simulink model "MX_Gateway"  (Simulink 9.0)
settings: Solver=FixedStepDiscrete  StartTime=0.0  StopTime=100  FixedStep=0.01  RelTol=1e-3
values from neighbouring files (symbols this model uses):
  ACTIVE = Bobcat.Parameter; CreateDefine( ACTIVE                         ,1                          ,'Define' ,'defines.h' )    [defines.m]
  ACTIVE_MODE_ONE = Bobcat.Parameter; CreateDefine( ACTIVE_MODE_ONE                ,19                         ,'Define' ,'defines.h' )    [defines.m]
  AUTHENTICATED = Bobcat.Parameter; CreateDefine( AUTHENTICATED                  ,1                          ,'Define' ,'defines.h' )    [defines.m]
  AUX_EMEA_CONTROLS = Bobcat.Parameter; CreateDefine( AUX_EMEA_CONTROLS  ,1   ,'Define' ,'defines.h' )    [enums.m]
  AUX_FLOW_HIGH = Bobcat.Parameter; CreateDefine( AUX_FLOW_HIGH           ,4  ,'Define' ,'defines.h' )    [enums.m]
  AUX_FLOW_INACTIVE = Bobcat.Parameter; CreateDefine( AUX_FLOW_INACTIVE       ,0  ,'Define' ,'defines.h' )    [enums.m]
  AUX_FLOW_LOW = Bobcat.Parameter; CreateDefine( AUX_FLOW_LOW            ,1  ,'Define' ,'defines.h' )    [enums.m]
  AUX_FLOW_MEDIUM = Bobcat.Parameter; CreateDefine( AUX_FLOW_MEDIUM         ,2  ,'Define' ,'defines.h' )    [enums.m]
  AUX_FLOW_STANDARD = Bobcat.Parameter; CreateDefine( AUX_FLOW_STANDARD       ,3  ,'Define' ,'defines.h' )    [enums.m]
  AUX_NON_FUNCTIONAL = Bobcat.Parameter; CreateDefine( AUX_NON_FUNCTIONAL ,255 ,'Define' ,'defines.h' )    [enums.m]
  BASE_ACTIVE = Bobcat.Parameter; CreateDefine( BASE_ACTIVE                    ,253                        ,'Define' ,'defines.h' )    [defines.m]
  BASE_DETENT = Bobcat.Parameter; CreateDefine( BASE_DETENT                    ,1                          ,'Define' ,'defines.h' )    [defines.m]
  BEEP_NONE = Bobcat.Parameter; CreateDefine( BEEP_NONE                      ,255                        ,'Define' ,'defines.h' )    [defines.m]
  CD_AIR_FILTER_DEBOUNCE_NORMAL = Bobcat.Parameter; CreateAliasCD( CD_AIR_FILTER_DEBOUNCE_NORMAL                        ,uint16(500)                ,'ConstantDataPointer' ,'CONSTANT_DATA' ,'AIR_FILTER_DEBOUNCE_NORMAL')    [cdata.m]    (also in hc12_cdata.m; m4_cdata.m)
  CD_AIR_FILTER_DEBOUNCE_NOT_CONNECTED = Bobcat.Parameter; CreateAliasCD( CD_AIR_FILTER_DEBOUNCE_NOT_CONNECTED                 ,uint16(3000)               ,'ConstantDataPointer' ,'CONSTANT_DATA' ,'AIR_FILTER_DEBOUNCE_NOT_CONNECTED')    [cdata.m]    (also in hc12_cdata.m; m4_cdata.m)
  CD_AIR_FILTER_DEBOUNCE_PLUGGED = Bobcat.Parameter; CreateAliasCD( CD_AIR_FILTER_DEBOUNCE_PLUGGED                       ,uint16(3000)               ,'ConstantDataPointer' ,'CONSTANT_DATA' ,'AIR_FILTER_DEBOUNCE_PLUGGED')    [cdata.m]    (also in hc12_cdata.m; m4_cdata.m)
  CD_AIR_FILTER_FUNCTION = Bobcat.Parameter; CreateAliasCD( CD_AIR_FILTER_FUNCTION                               ,uint8(255)                 ,'ConstantDataPointer' ,'CONSTANT_DATA' ,'AIR_FILTER_FUNCTION')    [cdata.m]    (also in hc12_cdata.m; m4_cdata.m)
  CD_AIR_FILTER_PLUGGED_WEIGHT = Bobcat.Parameter; CreateAliasCD( CD_AIR_FILTER_PLUGGED_WEIGHT                         ,uint8(2)                   ,'ConstantDataPointer' ,'CONSTANT_DATA' ,'AIR_FILTER_PLUGGED_WEIGHT')    [cdata.m]    (also in hc12_cdata.m; m4_cdata.m)
  CD_AIR_FILTER_UNPLUGGED_WEIGHT = Bobcat.Parameter; CreateAliasCD( CD_AIR_FILTER_UNPLUGGED_WEIGHT                       ,uint8(1)                   ,'ConstantDataPointer' ,'CONSTANT_DATA' ,'AIR_FILTER_UNPLUGGED_WEIGHT')    [cdata.m]    (also in hc12_cdata.m; m4_cdata.m)
  CD_AUTOIDLE_ARMBAR_DELAY = Bobcat.Parameter; CreateAliasCD( CD_AUTOIDLE_ARMBAR_DELAY                             ,uint8(2)                   ,'ConstantDataPointer' ,'CONSTANT_DATA' ,'AUTOIDLE_ARMBAR_DELAY')    [cdata.m]    (also in hc12_cdata.m; m4_cdata.m)
  CD_AUTOIDLE_FUNCTION = Bobcat.Parameter; CreateAliasCD( CD_AUTOIDLE_FUNCTION                                 ,uint8(255)                 ,'ConstantDataPointer' ,'CONSTANT_DATA' ,'AUTOIDLE_FUNCTION')    [cdata.m]    (also in hc12_cdata.m; m4_cdata.m)
  CD_AUTOIDLE_IDLE_DELAY = Bobcat.Parameter; CreateAliasCD( CD_AUTOIDLE_IDLE_DELAY                               ,uint8(40)                  ,'ConstantDataPointer' ,'CONSTANT_DATA' ,'AUTOIDLE_IDLE_DELAY')    [cdata.m]    (also in hc12_cdata.m; m4_cdata.m)
  CD_AUTOIDLE_LOW_IDLE_START = Bobcat.Parameter; CreateAliasCD( CD_AUTOIDLE_LOW_IDLE_START                           ,uint8(255)                 ,'ConstantDataPointer' ,'CONSTANT_DATA' ,'AUTOIDLE_LOW_IDLE_START')    [cdata.m]    (also in hc12_cdata.m; m4_cdata.m)
  CD_AUTOIDLE_PRESSURE_OFF = Bobcat.Parameter; CreateAliasCD( CD_AUTOIDLE_PRESSURE_OFF                             ,uint16(300)                ,'ConstantDataPointer' ,'CONSTANT_DATA' ,'AUTOIDLE_PRESSURE_OFF')    [cdata.m]    (also in hc12_cdata.m; m4_cdata.m)
  CD_AUTOIDLE_PRESSURE_ON = Bobcat.Parameter; CreateAliasCD( CD_AUTOIDLE_PRESSURE_ON                              ,uint16(270)                ,'ConstantDataPointer' ,'CONSTANT_DATA' ,'AUTOIDLE_PRESSURE_ON')    [cdata.m]    (also in hc12_cdata.m; m4_cdata.m)
  CD_AUTOSHIFT_FUNCTION = Bobcat.Parameter; CreateAliasCD( CD_AUTOSHIFT_FUNCTION                                ,uint8(255)                 ,'ConstantDataPointer' ,'CONSTANT_DATA' ,'AUTOSHIFT_FUNCTION')    [cdata.m]    (also in hc12_cdata.m; m4_cdata.m)
  CD_AUTOSHIFT_PHIGH1 = Bobcat.Parameter; CreateAliasCD( CD_AUTOSHIFT_PHIGH1                                  ,uint16(205)                ,'ConstantDataPointer' ,'CONSTANT_DATA' ,'AUTOSHIFT_PHIGH1')    [cdata.m]    (also in hc12_cdata.m; m4_cdata.m)
  CD_AUTOSHIFT_PHIGH2 = Bobcat.Parameter; CreateAliasCD( CD_AUTOSHIFT_PHIGH2                                  ,uint16(193)                ,'ConstantDataPointer' ,'CONSTANT_DATA' ,'AUTOSHIFT_PHIGH2')    [cdata.m]    (also in hc12_cdata.m; m4_cdata.m)
  CD_AUTOSHIFT_PHIGH_TEMP1 = Bobcat.Parameter; CreateAliasCD( CD_AUTOSHIFT_PHIGH_TEMP1                             ,int16(70)                  ,'ConstantDataPointer' ,'CONSTANT_DATA' ,'AUTOSHIFT_PHIGH_TEMP1')    [cdata.m]    (also in hc12_cdata.m; m4_cdata.m)
  CD_AUTOSHIFT_PHIGH_TEMP2 = Bobcat.Parameter; CreateAliasCD( CD_AUTOSHIFT_PHIGH_TEMP2                             ,int16(160)                 ,'ConstantDataPointer' ,'CONSTANT_DATA' ,'AUTOSHIFT_PHIGH_TEMP2')    [cdata.m]    (also in hc12_cdata.m; m4_cdata.m)
  CD_AUTOSHIFT_PLOW1 = Bobcat.Parameter; CreateAliasCD( CD_AUTOSHIFT_PLOW1                                   ,uint16(130)                ,'ConstantDataPointer' ,'CONSTANT_DATA' ,'AUTOSHIFT_PLOW1')    [cdata.m]    (also in hc12_cdata.m; m4_cdata.m)
  CD_AUTOSHIFT_PLOW2 = Bobcat.Parameter; CreateAliasCD( CD_AUTOSHIFT_PLOW2                                   ,uint16(100)                ,'ConstantDataPointer' ,'CONSTANT_DATA' ,'AUTOSHIFT_PLOW2')    [cdata.m]    (also in hc12_cdata.m; m4_cdata.m)
  CD_AUTOSHIFT_PLOW_TEMP1 = Bobcat.Parameter; CreateAliasCD( CD_AUTOSHIFT_PLOW_TEMP1                              ,int16(20)                  ,'ConstantDataPointer' ,'CONSTANT_DATA' ,'AUTOSHIFT_PLOW_TEMP1')    [cdata.m]    (also in hc12_cdata.m; m4_cdata.m)
  CD_AUTOSHIFT_PLOW_TEMP2 = Bobcat.Parameter; CreateAliasCD( CD_AUTOSHIFT_PLOW_TEMP2                              ,int16(80)                  ,'ConstantDataPointer' ,'CONSTANT_DATA' ,'AUTOSHIFT_PLOW_TEMP2')    [cdata.m]    (also in hc12_cdata.m; m4_cdata.m)
  CD_AUTOSHIFT_TRAVEL_DELAY = Bobcat.Parameter; CreateAliasCD( CD_AUTOSHIFT_TRAVEL_DELAY                            ,uint8(32)                  ,'ConstantDataPointer' ,'CONSTANT_DATA' ,'AUTOSHIFT_TRAVEL_DELAY')    [cdata.m]    (also in hc12_cdata.m; m4_cdata.m)
  CD_AUX2_CRACKPOINT_CURRENT = Bobcat.Parameter; CreateAliasCD( CD_AUX2_CRACKPOINT_CURRENT                           ,fi(0,0,16,12,'hex','0c28') ,'ConstantDataPointer' ,'CONSTANT_DATA' ,'AUX2_CRACKPOINT_CURRENT')    [cdata.m]    (also in hc12_cdata.m; m4_cdata.m)
  CD_AUX2_FLOW_LOW_FUNCTION = Bobcat.Parameter; CreateAliasCD( CD_AUX2_FLOW_LOW_FUNCTION                            ,uint8(255)                 ,'ConstantDataPointer' ,'CONSTANT_DATA' ,'AUX2_FLOW_LOW_FUNCTION')    [cdata.m]    (also in hc12_cdata.m; m4_cdata.m)
  CD_AUX2_FLOW_MED_FUNCTION = Bobcat.Parameter; CreateAliasCD( CD_AUX2_FLOW_MED_FUNCTION                            ,uint8(255)                 ,'ConstantDataPointer' ,'CONSTANT_DATA' ,'AUX2_FLOW_MED_FUNCTION')    [cdata.m]    (also in hc12_cdata.m; m4_cdata.m)
  CD_AUX2_HI_FLOW_PERCENT = Bobcat.Parameter; CreateAliasCD( CD_AUX2_HI_FLOW_PERCENT                              ,fi(0,0,16,16,'hex','ffff') ,'ConstantDataPointer' ,'CONSTANT_DATA' ,'AUX2_HI_FLOW_PERCENT')    [cdata.m]    (also in hc12_cdata.m; m4_cdata.m)
  CD_AUX2_LOW_FLOW_PERCENT = Bobcat.Parameter; CreateAliasCD( CD_AUX2_LOW_FLOW_PERCENT                             ,fi(0,0,16,16,'hex','7333') ,'ConstantDataPointer' ,'CONSTANT_DATA' ,'AUX2_LOW_FLOW_PERCENT')    [cdata.m]    (also in hc12_cdata.m; m4_cdata.m)
  CD_AUX2_MAX_FLOW_CURRENT = Bobcat.Parameter; CreateAliasCD( CD_AUX2_MAX_FLOW_CURRENT                             ,fi(0,0,16,12,'hex','14cc') ,'ConstantDataPointer' ,'CONSTANT_DATA' ,'AUX2_MAX_FLOW_CURRENT')    [cdata.m]    (also in hc12_cdata.m; m4_cdata.m)
  CD_AUX2_MED_FLOW_PERCENT = Bobcat.Parameter; CreateAliasCD( CD_AUX2_MED_FLOW_PERCENT                             ,fi(0,0,16,16,'hex','9999') ,'ConstantDataPointer' ,'CONSTANT_DATA' ,'AUX2_MED_FLOW_PERCENT')    [cdata.m]    (also in hc12_cdata.m; m4_cdata.m)
  CD_AUX_CRACKPOINT_CURRENT = Bobcat.Parameter; CreateAliasCD( CD_AUX_CRACKPOINT_CURRENT                            ,fi(0,0,16,12,'hex','0000') ,'ConstantDataPointer' ,'CONSTANT_DATA' ,'AUX_CRACKPOINT_CURRENT')    [cdata.m]    (also in hc12_cdata.m; m4_cdata.m)
  CD_AUX_DETENT_FLASH_TIME = Bobcat.Parameter; CreateAliasCD( CD_AUX_DETENT_FLASH_TIME                             ,uint16(72)                 ,'ConstantDataPointer' ,'CONSTANT_DATA' ,'AUX_DETENT_FLASH_TIME')    [cdata.m]    (also in hc12_cdata.m; m4_cdata.m)
  CD_AUX_DETENT_HOLD_TIME = Bobcat.Parameter; CreateAliasCD( CD_AUX_DETENT_HOLD_TIME                              ,uint16(100)                ,'ConstantDataPointer' ,'CONSTANT_DATA' ,'AUX_DETENT_HOLD_TIME')    [cdata.m]    (also in hc12_cdata.m; m4_cdata.m)
  CD_AUX_FULL_ON_AUX1 = Bobcat.Parameter; CreateAliasCD( CD_AUX_FULL_ON_AUX1                                  ,fi(0,0,16,12,'hex','10cd') ,'ConstantDataPointer' ,'CONSTANT_DATA' ,'AUX_FULL_ON_AUX1')    [cdata.m]    (also in hc12_cdata.m; m4_cdata.m)
  CD_AUX_FULL_ON_AUX2 = Bobcat.Parameter; CreateAliasCD( CD_AUX_FULL_ON_AUX2                                  ,fi(0,0,16,12,'hex','139d') ,'ConstantDataPointer' ,'CONSTANT_DATA' ,'AUX_FULL_ON_AUX2')    [cdata.m]    (also in hc12_cdata.m; m4_cdata.m)
  CD_AUX_FULL_ON_AUX3 = Bobcat.Parameter; CreateAliasCD( CD_AUX_FULL_ON_AUX3                                  ,fi(0,0,16,12,'hex','17d7') ,'ConstantDataPointer' ,'CONSTANT_DATA' ,'AUX_FULL_ON_AUX3')    [cdata.m]    (also in hc12_cdata.m; m4_cdata.m)
  CD_AUX_FUNCTION = Bobcat.Parameter; CreateAliasCD( CD_AUX_FUNCTION                                      ,uint8(0)                   ,'ConstantDataPointer' ,'CONSTANT_DATA' ,'AUX_FUNCTION')    [cdata.m]    (also in hc12_cdata.m; m4_cdata.m)
  CD_AUX_RATE_LIMIT_CRACKPOINT = Bobcat.Parameter; CreateAliasCD( CD_AUX_RATE_LIMIT_CRACKPOINT                         ,fi(0,0,16,12,'hex','0800') ,'ConstantDataPointer' ,'CONSTANT_DATA' ,'AUX_RATE_LIMIT_CRACKPOINT')    [cdata.m]    (also in hc12_cdata.m; m4_cdata.m)
  CD_AUX_RATE_LIMIT_CRACKPOINT_RATE = Bobcat.Parameter; CreateAliasCD( CD_AUX_RATE_LIMIT_CRACKPOINT_RATE                    ,fi(0,1,32,12,'int',102400) ,'ConstantDataPointer' ,'CONSTANT_DATA' ,'AUX_RATE_LIMIT_CRACKPOINT_RATE')    [cdata.m]    (also in hc12_cdata.m; m4_cdata.m)
  CD_AUX_RATE_LIMIT_FUNCTION = Bobcat.Parameter; CreateAliasCD( CD_AUX_RATE_LIMIT_FUNCTION                           ,uint8(255)                 ,'ConstantDataPointer' ,'CONSTANT_DATA' ,'AUX_RATE_LIMIT_FUNCTION')    [cdata.m]    (also in hc12_cdata.m; m4_cdata.m)
  CD_AUX_RATE_LIMIT_MAX_ENG_SPD = Bobcat.Parameter; CreateAliasCD( CD_AUX_RATE_LIMIT_MAX_ENG_SPD                        ,uint16(1600)               ,'ConstantDataPointer' ,'CONSTANT_DATA' ,'AUX_RATE_LIMIT_MAX_ENG_SPD')    [cdata.m]    (also in hc12_cdata.m; m4_cdata.m)
  CD_AUX_RATE_LIMIT_MIN_ENG_SPD = Bobcat.Parameter; CreateAliasCD( CD_AUX_RATE_LIMIT_MIN_ENG_SPD                        ,uint16(100)                ,'ConstantDataPointer' ,'CONSTANT_DATA' ,'AUX_RATE_LIMIT_MIN_ENG_SPD')    [cdata.m]    (also in hc12_cdata.m; m4_cdata.m)
  CD_AUX_RATE_LIMIT_NORMAL_RATE = Bobcat.Parameter; CreateAliasCD( CD_AUX_RATE_LIMIT_NORMAL_RATE                        ,fi(0,1,32,12,'int',2048)   ,'ConstantDataPointer' ,'CONSTANT_DATA' ,'AUX_RATE_LIMIT_NORMAL_RATE')    [cdata.m]    (also in hc12_cdata.m; m4_cdata.m)
  CD_AUXPOT_CAL_DEADBAND_HIGH = Bobcat.Parameter; CreateAliasCD( CD_AUXPOT_CAL_DEADBAND_HIGH                          ,uint16(574)                ,'ConstantDataPointer' ,'CONSTANT_DATA' ,'AUXPOT_CAL_DEADBAND_HIGH')    [cdata.m]    (also in hc12_cdata.m; m4_cdata.m)
  CD_AUXPOT_CAL_DEADBAND_LOW = Bobcat.Parameter; CreateAliasCD( CD_AUXPOT_CAL_DEADBAND_LOW                           ,uint16(424)                ,'ConstantDataPointer' ,'CONSTANT_DATA' ,'AUXPOT_CAL_DEADBAND_LOW')    [cdata.m]    (also in hc12_cdata.m; m4_cdata.m)
  CD_AUXPOT_CENTER_RATIO = Bobcat.Parameter; CreateAliasCD( CD_AUXPOT_CENTER_RATIO                               ,uint16(500)                ,'ConstantDataPointer' ,'CONSTANT_DATA' ,'AUXPOT_CENTER_RATIO')    [cdata.m]    (also in hc12_cdata.m; m4_cdata.m)
  CD_AUXPOT_DEADBAND = Bobcat.Parameter; CreateAliasCD( CD_AUXPOT_DEADBAND                                   ,uint8(38)                  ,'ConstantDataPointer' ,'CONSTANT_DATA' ,'AUXPOT_DEADBAND')    [cdata.m]    (also in hc12_cdata.m; m4_cdata.m)
  CD_AUXPOT_FUNCTION = Bobcat.Parameter; CreateAliasCD( CD_AUXPOT_FUNCTION                                   ,uint8(0)                   ,'ConstantDataPointer' ,'CONSTANT_DATA' ,'AUXPOT_FUNCTION')    [cdata.m]    (also in hc12_cdata.m; m4_cdata.m)
  CD_AUXPOT_RATIO_GAIN = Bobcat.Parameter; CreateAliasCD( CD_AUXPOT_RATIO_GAIN                                 ,uint16(1000)               ,'ConstantDataPointer' ,'CONSTANT_DATA' ,'AUXPOT_RATIO_GAIN')    [cdata.m]    (also in hc12_cdata.m; m4_cdata.m)
  CD_CAN_THROTTLE_FUNCTION = Bobcat.Parameter; CreateAliasCD( CD_CAN_THROTTLE_FUNCTION                             ,uint8(255)                 ,'ConstantDataPointer' ,'CONSTANT_DATA' ,'CAN_THROTTLE_FUNCTION')    [cdata.m]    (also in hc12_cdata.m; m4_cdata.m)
  CD_CAN_THROTTLE_REVERSE = Bobcat.Parameter; CreateAliasCD( CD_CAN_THROTTLE_REVERSE                              ,uint8(255)                 ,'ConstantDataPointer' ,'CONSTANT_DATA' ,'CAN_THROTTLE_REVERSE')    [cdata.m]    (also in hc12_cdata.m; m4_cdata.m)
  CD_CONSOLE_DEBOUNCE_ABSENT = Bobcat.Parameter; CreateAliasCD( CD_CONSOLE_DEBOUNCE_ABSENT                           ,uint16(12)                 ,'ConstantDataPointer' ,'CONSTANT_DATA' ,'CONSOLE_DEBOUNCE_ABSENT')    [cdata.m]    (also in hc12_cdata.m; m4_cdata.m)
  CD_CONSOLE_DEBOUNCE_OORH = Bobcat.Parameter; CreateAliasCD( CD_CONSOLE_DEBOUNCE_OORH                             ,uint16(12)                 ,'ConstantDataPointer' ,'CONSTANT_DATA' ,'CONSOLE_DEBOUNCE_OORH')    [cdata.m]    (also in hc12_cdata.m; m4_cdata.m)
  CD_CONSOLE_DEBOUNCE_OORL = Bobcat.Parameter; CreateAliasCD( CD_CONSOLE_DEBOUNCE_OORL                             ,uint16(12)                 ,'ConstantDataPointer' ,'CONSTANT_DATA' ,'CONSOLE_DEBOUNCE_OORL')    [cdata.m]    (also in hc12_cdata.m; m4_cdata.m)
  CD_CONSOLE_DEBOUNCE_PRESENT = Bobcat.Parameter; CreateAliasCD( CD_CONSOLE_DEBOUNCE_PRESENT                          ,uint16(12)                 ,'ConstantDataPointer' ,'CONSTANT_DATA' ,'CONSOLE_DEBOUNCE_PRESENT')    [cdata.m]    (also in hc12_cdata.m; m4_cdata.m)
  CD_CONSOLE_FUNCTION = Bobcat.Parameter; CreateAliasCD( CD_CONSOLE_FUNCTION                                  ,uint8(0)                   ,'ConstantDataPointer' ,'CONSTANT_DATA' ,'CONSOLE_FUNCTION')    [cdata.m]    (also in hc12_cdata.m; m4_cdata.m)
  CD_CONSOLE_OORH = Bobcat.Parameter; CreateAliasCD( CD_CONSOLE_OORH                                      ,uint16(740)                ,'ConstantDataPointer' ,'CONSTANT_DATA' ,'CONSOLE_OORH')    [cdata.m]    (also in hc12_cdata.m; m4_cdata.m)
  CD_CONSOLE_OORL = Bobcat.Parameter; CreateAliasCD( CD_CONSOLE_OORL                                      ,uint16(10)                 ,'ConstantDataPointer' ,'CONSTANT_DATA' ,'CONSOLE_OORL')    [cdata.m]    (also in hc12_cdata.m; m4_cdata.m)
  CD_CONSOLE_PRESENT_HIGH = Bobcat.Parameter; CreateAliasCD( CD_CONSOLE_PRESENT_HIGH                              ,uint16(515)                ,'ConstantDataPointer' ,'CONSTANT_DATA' ,'CONSOLE_PRESENT_HIGH')    [cdata.m]    (also in hc12_cdata.m; m4_cdata.m)
  CD_CONSOLE_PRESENT_LOW = Bobcat.Parameter; CreateAliasCD( CD_CONSOLE_PRESENT_LOW                               ,uint16(375)                ,'ConstantDataPointer' ,'CONSTANT_DATA' ,'CONSOLE_PRESENT_LOW')    [cdata.m]    (also in hc12_cdata.m; m4_cdata.m)
  CD_DELUXE_GEN5_5_FUNCTION = Bobcat.Parameter; CreateAliasCD( CD_DELUXE_GEN5_5_FUNCTION                            ,uint8(255)                 ,'ConstantDataPointer' ,'CONSTANT_DATA' ,'DELUXE_GEN5_5_FUNCTION')    [cdata.m]    (also in hc12_cdata.m; m4_cdata.m)
  CD_DELUXE_GEN5_FUNCTION = Bobcat.Parameter; CreateAliasCD( CD_DELUXE_GEN5_FUNCTION                              ,uint8(255)                 ,'ConstantDataPointer' ,'CONSTANT_DATA' ,'DELUXE_GEN5_FUNCTION')    [cdata.m]    (also in hc12_cdata.m; m4_cdata.m)
  CD_DERATE_ENGINE_SPEED_DEBOUNCE_HIGH = Bobcat.Parameter; CreateAliasCD( CD_DERATE_ENGINE_SPEED_DEBOUNCE_HIGH                 ,uint16(200)                ,'ConstantDataPointer' ,'CONSTANT_DATA' ,'DERATE_ENGINE_SPEED_DEBOUNCE_HIGH')    [cdata.m]    (also in hc12_cdata.m; m4_cdata.m)
  CD_DERATE_ENGINE_SPEED_DEBOUNCE_SHUTDOWN = Bobcat.Parameter; CreateAliasCD( CD_DERATE_ENGINE_SPEED_DEBOUNCE_SHUTDOWN             ,uint16(1000)               ,'ConstantDataPointer' ,'CONSTANT_DATA' ,'DERATE_ENGINE_SPEED_DEBOUNCE_SHUTDOWN')    [cdata.m]    (also in hc12_cdata.m; m4_cdata.m)
  CD_DERATE_ENGINE_SPEED_HIGH_OFFSET = Bobcat.Parameter; CreateAliasCD( CD_DERATE_ENGINE_SPEED_HIGH_OFFSET                   ,uint16(200)                ,'ConstantDataPointer' ,'CONSTANT_DATA' ,'DERATE_ENGINE_SPEED_HIGH_OFFSET')    [cdata.m]    (also in hc12_cdata.m; m4_cdata.m)
  CD_DERATE_METHOD = Bobcat.Parameter; CreateAliasCD( CD_DERATE_METHOD                                     ,uint8(0)                   ,'ConstantDataPointer' ,'CONSTANT_DATA' ,'DERATE_METHOD')    [cdata.m]    (also in hc12_cdata.m; m4_cdata.m)
  CD_DUMP_VALVE_FUNCTION = Bobcat.Parameter; CreateAliasCD( CD_DUMP_VALVE_FUNCTION                               ,uint8(255)                 ,'ConstantDataPointer' ,'CONSTANT_DATA' ,'DUMP_VALVE_FUNCTION')    [cdata.m]    (also in hc12_cdata.m; m4_cdata.m)
  CD_DUMP_VALVE_ON_CURRENT = Bobcat.Parameter; CreateAliasCD( CD_DUMP_VALVE_ON_CURRENT                             ,fi(0,0,16,12,'hex','ffff') ,'ConstantDataPointer' ,'CONSTANT_DATA' ,'DUMP_VALVE_ON_CURRENT')    [cdata.m]    (also in hc12_cdata.m; m4_cdata.m)
  CD_ECO_MODE_THROTTLE_REDUCTION = Bobcat.Parameter; CreateAliasCD( CD_ECO_MODE_THROTTLE_REDUCTION                       ,uint16(850)                ,'ConstantDataPointer' ,'CONSTANT_DATA' ,'ECO_MODE_THROTTLE_REDUCTION')    [cdata.m]    (also in hc12_cdata.m; m4_cdata.m)
  CD_ECT_FILTER_GAIN = Bobcat.Parameter; CreateAliasCD( CD_ECT_FILTER_GAIN                                   ,uint8(6)                   ,'ConstantDataPointer' ,'CONSTANT_DATA' ,'ECT_FILTER_GAIN')    [cdata.m]    (also in hc12_cdata.m; m4_cdata.m)
  CD_ECU_FUNCTION = Bobcat.Parameter; CreateAliasCD( CD_ECU_FUNCTION                                      ,uint8(255)                 ,'ConstantDataPointer' ,'CONSTANT_DATA' ,'ECU_FUNCTION')    [cdata.m]    (also in hc12_cdata.m; m4_cdata.m)
  CD_ECU_HOLD_DEBOUNCE_ERROR_ON = Bobcat.Parameter; CreateAliasCD( CD_ECU_HOLD_DEBOUNCE_ERROR_ON                        ,uint16(20)                 ,'ConstantDataPointer' ,'CONSTANT_DATA' ,'ECU_HOLD_DEBOUNCE_ERROR_ON')    [cdata.m]    (also in hc12_cdata.m; m4_cdata.m)
  CD_EMEA_CONTROLS_HOLD_TIME = Bobcat.Parameter; CreateAliasCD( CD_EMEA_CONTROLS_HOLD_TIME                           ,uint16(30)                 ,'ConstantDataPointer' ,'CONSTANT_DATA' ,'EMEA_CONTROLS_HOLD_TIME')    [cdata.m]    (also in hc12_cdata.m; m4_cdata.m)
  CD_EMEA_CONTROLS_OPTION_A = Bobcat.Parameter; CreateAliasCD( CD_EMEA_CONTROLS_OPTION_A                            ,uint8(255)                 ,'ConstantDataPointer' ,'CONSTANT_DATA' ,'EMEA_CONTROLS_OPTION_A')    [cdata.m]    (also in hc12_cdata.m; m4_cdata.m)
  CD_EMEA_CONTROLS_OPTION_C = Bobcat.Parameter; CreateAliasCD( CD_EMEA_CONTROLS_OPTION_C                            ,uint8(255)                 ,'ConstantDataPointer' ,'CONSTANT_DATA' ,'EMEA_CONTROLS_OPTION_C')    [cdata.m]    (also in hc12_cdata.m; m4_cdata.m)
  CD_EMEA_CONTROLS_REVERSE = Bobcat.Parameter; CreateAliasCD( CD_EMEA_CONTROLS_REVERSE                             ,uint8(255)                 ,'ConstantDataPointer' ,'CONSTANT_DATA' ,'EMEA_CONTROLS_REVERSE')    [cdata.m]    (also in hc12_cdata.m; m4_cdata.m)
  CD_EMEA_ELECTRIC_VERSION = Bobcat.Parameter; CreateAliasCD( CD_EMEA_ELECTRIC_VERSION                             ,uint8(0)                   ,'ConstantDataPointer' ,'CONSTANT_DATA' ,'EMEA_ELECTRIC_VERSION')    [cdata.m]    (also in hc12_cdata.m; m4_cdata.m)
  CD_EMEA_POT_CAL_DEADBAND_HIGH = Bobcat.Parameter; CreateAliasCD( CD_EMEA_POT_CAL_DEADBAND_HIGH                        ,uint16(574)                ,'ConstantDataPointer' ,'CONSTANT_DATA' ,'EMEA_POT_CAL_DEADBAND_HIGH')    [cdata.m]    (also in hc12_cdata.m; m4_cdata.m)
  CD_EMEA_POT_CAL_DEADBAND_LOW = Bobcat.Parameter; CreateAliasCD( CD_EMEA_POT_CAL_DEADBAND_LOW                         ,uint16(424)                ,'ConstantDataPointer' ,'CONSTANT_DATA' ,'EMEA_POT_CAL_DEADBAND_LOW')    [cdata.m]    (also in hc12_cdata.m; m4_cdata.m)
  CD_ENGINE_RPM_FACTOR = Bobcat.Parameter; CreateAliasCD( CD_ENGINE_RPM_FACTOR                                 ,uint8(35)                  ,'ConstantDataPointer' ,'CONSTANT_DATA' ,'ENGINE_RPM_FACTOR')    [cdata.m]    (also in hc12_cdata.m; m4_cdata.m)
  CD_ENGINE_SPEED_FILTER_FAST_VALUE = Bobcat.Parameter; CreateAliasCD( CD_ENGINE_SPEED_FILTER_FAST_VALUE                    ,uint8(1)                   ,'ConstantDataPointer' ,'CONSTANT_DATA' ,'ENGINE_SPEED_FILTER_FAST_VALUE')    [cdata.m]    (also in hc12_cdata.m; m4_cdata.m)
  CD_ENGINE_SPEED_FILTER_SLOW_VALUE = Bobcat.Parameter; CreateAliasCD( CD_ENGINE_SPEED_FILTER_SLOW_VALUE                    ,uint8(4)                   ,'ConstantDataPointer' ,'CONSTANT_DATA' ,'ENGINE_SPEED_FILTER_SLOW_VALUE')    [cdata.m]    (also in hc12_cdata.m; m4_cdata.m)
  CD_ENGINE_SPEED_RUNNING = Bobcat.Parameter; CreateAliasCD( CD_ENGINE_SPEED_RUNNING                              ,uint16(800)                ,'ConstantDataPointer' ,'CONSTANT_DATA' ,'ENGINE_SPEED_RUNNING')    [cdata.m]    (also in hc12_cdata.m; m4_cdata.m)
  CD_ENGINE_START_DELAY_DEBOUNCE_RPM_NO_SIGNAL = Bobcat.Parameter; CreateAliasCD( CD_ENGINE_START_DELAY_DEBOUNCE_RPM_NO_SIGNAL         ,uint16(0)                  ,'ConstantDataPointer' ,'CONSTANT_DATA' ,'ENGINE_START_DELAY_DEBOUNCE_RPM_NO_SIGNAL')    [cdata.m]    (also in hc12_cdata.m; m4_cdata.m)
  CD_ENGINE_START_DELAY_OFF_TIME = Bobcat.Parameter; CreateAliasCD( CD_ENGINE_START_DELAY_OFF_TIME                       ,uint16(0)                  ,'ConstantDataPointer' ,'CONSTANT_DATA' ,'ENGINE_START_DELAY_OFF_TIME')    [cdata.m]    (also in hc12_cdata.m; m4_cdata.m)
  CD_ENGINE_START_DELAY_RPM_NO_SIGNAL = Bobcat.Parameter; CreateAliasCD( CD_ENGINE_START_DELAY_RPM_NO_SIGNAL                  ,uint16(0)                  ,'ConstantDataPointer' ,'CONSTANT_DATA' ,'ENGINE_START_DELAY_RPM_NO_SIGNAL')    [cdata.m]    (also in hc12_cdata.m; m4_cdata.m)
  CD_EOP_DEBOUNCE_EXTREMELY_LOW = Bobcat.Parameter; CreateAliasCD( CD_EOP_DEBOUNCE_EXTREMELY_LOW                        ,uint16(50)                 ,'ConstantDataPointer' ,'CONSTANT_DATA' ,'EOP_DEBOUNCE_EXTREMELY_LOW')    [cdata.m]    (also in hc12_cdata.m; m4_cdata.m)
  CD_EOP_DEBOUNCE_NORMAL = Bobcat.Parameter; CreateAliasCD( CD_EOP_DEBOUNCE_NORMAL                               ,uint16(50)                 ,'ConstantDataPointer' ,'CONSTANT_DATA' ,'EOP_DEBOUNCE_NORMAL')    [cdata.m]    (also in hc12_cdata.m; m4_cdata.m)
  CD_EOP_DEBOUNCE_SHUTDOWN = Bobcat.Parameter; CreateAliasCD( CD_EOP_DEBOUNCE_SHUTDOWN                             ,uint16(100)                ,'ConstantDataPointer' ,'CONSTANT_DATA' ,'EOP_DEBOUNCE_SHUTDOWN')    [cdata.m]    (also in hc12_cdata.m; m4_cdata.m)
  CD_EOP_FILTER_VALUE = Bobcat.Parameter; CreateAliasCD( CD_EOP_FILTER_VALUE                                  ,uint8(3)                   ,'ConstantDataPointer' ,'CONSTANT_DATA' ,'EOP_FILTER_VALUE')    [cdata.m]    (also in hc12_cdata.m; m4_cdata.m)
  CD_EOP_FUNCTION = Bobcat.Parameter; CreateAliasCD( CD_EOP_FUNCTION                                      ,uint8(1)                   ,'ConstantDataPointer' ,'CONSTANT_DATA' ,'EOP_FUNCTION')    [cdata.m]    (also in hc12_cdata.m; m4_cdata.m)
  CD_EOP_SWITCH_GAUGE_VALUE = Bobcat.Parameter; CreateAliasCD( CD_EOP_SWITCH_GAUGE_VALUE                            ,uint16(125)                ,'ConstantDataPointer' ,'CONSTANT_DATA' ,'EOP_SWITCH_GAUGE_VALUE')    [cdata.m]    (also in hc12_cdata.m; m4_cdata.m)
  CD_EOP_SWITCH_LOW = Bobcat.Parameter; CreateAliasCD( CD_EOP_SWITCH_LOW                                    ,uint16(50)                 ,'ConstantDataPointer' ,'CONSTANT_DATA' ,'EOP_SWITCH_LOW')    [cdata.m]    (also in hc12_cdata.m; m4_cdata.m)
  CD_EOP_SWITCH_PRESSURE_VALUE = Bobcat.Parameter; CreateAliasCD( CD_EOP_SWITCH_PRESSURE_VALUE                         ,uint16(40)                 ,'ConstantDataPointer' ,'CONSTANT_DATA' ,'EOP_SWITCH_PRESSURE_VALUE')    [cdata.m]    (also in hc12_cdata.m; m4_cdata.m)
  CD_EOT_DEBOUNCE_EXTREMELY_LOW = Bobcat.Parameter; CreateAliasCD( CD_EOT_DEBOUNCE_EXTREMELY_LOW                        ,uint16(2)                  ,'ConstantDataPointer' ,'CONSTANT_DATA' ,'EOT_DEBOUNCE_EXTREMELY_LOW')    [cdata.m]    (also in hc12_cdata.m; m4_cdata.m)
  CD_EOT_DEBOUNCE_LOW = Bobcat.Parameter; CreateAliasCD( CD_EOT_DEBOUNCE_LOW                                  ,uint16(2)                  ,'ConstantDataPointer' ,'CONSTANT_DATA' ,'EOT_DEBOUNCE_LOW')    [cdata.m]    (also in hc12_cdata.m; m4_cdata.m)
  CD_EOT_DEBOUNCE_NORMAL = Bobcat.Parameter; CreateAliasCD( CD_EOT_DEBOUNCE_NORMAL                               ,uint16(2)                  ,'ConstantDataPointer' ,'CONSTANT_DATA' ,'EOT_DEBOUNCE_NORMAL')    [cdata.m]    (also in hc12_cdata.m; m4_cdata.m)
  CD_EOT_EXTREMELY_LOW = Bobcat.Parameter; CreateAliasCD( CD_EOT_EXTREMELY_LOW                                 ,uint16(81)                 ,'ConstantDataPointer' ,'CONSTANT_DATA' ,'EOT_EXTREMELY_LOW')    [cdata.m]    (also in hc12_cdata.m; m4_cdata.m)
  CD_EOT_FUNCTION = Bobcat.Parameter; CreateAliasCD( CD_EOT_FUNCTION                                      ,uint8(255)                 ,'ConstantDataPointer' ,'CONSTANT_DATA' ,'EOT_FUNCTION')    [cdata.m]    (also in hc12_cdata.m; m4_cdata.m)
  CD_EOT_LOW = Bobcat.Parameter; CreateAliasCD( CD_EOT_LOW                                           ,uint16(90)                 ,'ConstantDataPointer' ,'CONSTANT_DATA' ,'EOT_LOW')    [cdata.m]    (also in hc12_cdata.m; m4_cdata.m)
  CD_EOT_TEMPERATURE_HYSTERESIS = Bobcat.Parameter; CreateAliasCD( CD_EOT_TEMPERATURE_HYSTERESIS                        ,uint16(2)                  ,'ConstantDataPointer' ,'CONSTANT_DATA' ,'EOT_TEMPERATURE_HYSTERESIS')    [cdata.m]    (also in hc12_cdata.m; m4_cdata.m)
  CD_FAST_TRAVEL_FUNCTION = Bobcat.Parameter; CreateAliasCD( CD_FAST_TRAVEL_FUNCTION                              ,uint8(255)                 ,'ConstantDataPointer' ,'CONSTANT_DATA' ,'FAST_TRAVEL_FUNCTION')    [cdata.m]    (also in hc12_cdata.m; m4_cdata.m)
  CD_FAST_TRAVEL_THROTTLE_DELAY = Bobcat.Parameter; CreateAliasCD( CD_FAST_TRAVEL_THROTTLE_DELAY                        ,uint16(10)                 ,'ConstantDataPointer' ,'CONSTANT_DATA' ,'FAST_TRAVEL_THROTTLE_DELAY')    [cdata.m]    (also in hc12_cdata.m; m4_cdata.m)
  CD_FAST_TRAVEL_THROTTLE_LIMIT = Bobcat.Parameter; CreateAliasCD( CD_FAST_TRAVEL_THROTTLE_LIMIT                        ,uint16(1000)               ,'ConstantDataPointer' ,'CONSTANT_DATA' ,'FAST_TRAVEL_THROTTLE_LIMIT')    [cdata.m]    (also in hc12_cdata.m; m4_cdata.m)
  CD_FLYWHEEL_TEETH = Bobcat.Parameter; CreateAliasCD( CD_FLYWHEEL_TEETH                                    ,uint8(83)                  ,'ConstantDataPointer' ,'CONSTANT_DATA' ,'FLYWHEEL_TEETH')    [cdata.m]    (also in hc12_cdata.m; m4_cdata.m)
  CD_FUEL_LEVEL_FILTER_FAST_VALUE = Bobcat.Parameter; CreateAliasCD( CD_FUEL_LEVEL_FILTER_FAST_VALUE                      ,uint8(5)                   ,'ConstantDataPointer' ,'CONSTANT_DATA' ,'FUEL_LEVEL_FILTER_FAST_VALUE')    [cdata.m]    (also in hc12_cdata.m; m4_cdata.m)
  CD_FUEL_LEVEL_FILTER_SLOW_VALUE = Bobcat.Parameter; CreateAliasCD( CD_FUEL_LEVEL_FILTER_SLOW_VALUE                      ,uint8(10)                  ,'ConstantDataPointer' ,'CONSTANT_DATA' ,'FUEL_LEVEL_FILTER_SLOW_VALUE')    [cdata.m]    (also in hc12_cdata.m; m4_cdata.m)
  CD_FUEL_PRIMARY_FEEDBACK_FUNCTION = Bobcat.Parameter; CreateAliasCD( CD_FUEL_PRIMARY_FEEDBACK_FUNCTION                    ,uint8(0)                   ,'ConstantDataPointer' ,'CONSTANT_DATA' ,'FUEL_PRIMARY_FEEDBACK_FUNCTION')    [cdata.m]    (also in hc12_cdata.m; m4_cdata.m)
  ... (+246 more)
undefined symbols (not defined in the file or in its folder): sigbuilder_block
undefined symbols (not defined in the file): CD_FUEL_RESOLUTION_TIMER
libraries: simulink x186, MX_Library x107
(part 25 of 48)

== system MX_Gateway/MX_Gtwy_Control/Outputs/Aux_Hydraulics/Aux_Hydraulics/Aux_Hyd_Commands_Current_Control/Aux Table Select/EMEA_Control_AdjFlow ==
  note: The above method of reducing flow was implemented so that the existing
      | current versus pot position tables would not have to change.  The problem
      | with that method is that the relationship between flow versus current must
      | be linear (i.e. 50% of current/spool position is not necessarily 50% flow
      | depending on the design of the spool).
      |
      | The commented out method below would be better, but would require a
      | do-over on the tables.
  pot_Position : Inport
  flow_Percent : Inport  Port=2
  %Flow vs Pot Position : Lookup  Commented=on  InputValues=[0 200 300 450 500 550 700 800 1000]  Table=[-1 -.25 -.15 0 0 0 .15 .25 1]  LookUpMeth=Interpolation-Use End Values  OutDataTypeStr=fixdt(0,16,12)  SaturateOnIntegerOverflow=on
      settings: AttributesFormatString=input = %<InputValues>\noutput = %<OutputValues>
  Aux_Table3 : Lookup  InputValues=[20:6:980]  Table=MXGTW_AUXHYD3  LookUpMeth=Interpolation-Use End Values  OutDataTypeStr=fixdt(0,16,12)  SaturateOnIntegerOverflow=on
      settings: AttributesFormatString=input = %<InputValues>\noutput = %<OutputValues>
  Constant4 : Constant  Value=CD_AUX_CRACKPOINT_CURRENT  OutDataTypeStr=fixdt(0,16,12)
  Current versus %Flow : Lookup  Commented=on  InputValues=[-1:0.0125:1]  Table=MXGTW_OFFSET  LookUpMeth=Interpolation-Use End Values  OutDataTypeStr=fixdt(0,16,12)  SaturateOnIntegerOverflow=on
      settings: AttributesFormatString=input = %<InputValues>\noutput = %<OutputValues>
  Data Type Conversion : DataTypeConversion  OutDataTypeStr=fixdt(1,16,12)
      settings: AttributesFormatString=%<OutDataTypeStr>
  Data Type Conversion1 : DataTypeConversion  OutDataTypeStr=fixdt(0,16,12)
      settings: AttributesFormatString=%<OutDataTypeStr>
  Gain : Gain  Gain=fi(0.01,0,16,22)  ParamMin=0.0099  ParamMax=0.0101  ParamDataTypeStr=fixdt(0,16,22)  OutDataTypeStr=fixdt(0,16,16)
  Percent Flow : Constant  Commented=on  Value=0.5
  Pot position : Constant  Commented=on
  Product : Product  OutDataTypeStr=fixdt(0,16,16)
  Product1 : Product  Commented=on  OutDataTypeStr=fixdt(0,16,16)
  Saturation : Saturate  UpperLimit=2.0  LowerLimit=0.0
      settings: AttributesFormatString=|
      | Upper Limit = %<UpperLimit>
      | Lower Limit = %<LowerLimit>
  Sum : Sum  Inputs=-+|
  Sum1 : Sum  Inputs=++|
  Terminator : Terminator  Commented=on
  iCmd : Outport
  wires:
  pot_Position/1 -> Aux_Table3/1
  Sum/1 -> Saturation/1
  Data Type Conversion/1 -> Sum/2
  Constant4/1 -> Sum1/1, Sum/1
  Product/1 -> Sum1/2
  Saturation/1 -> Data Type Conversion1/1
  Data Type Conversion1/1 -> Product/1
  Aux_Table3/1 -> Data Type Conversion/1
  flow_Percent/1 -> Gain/1
  Sum1/1 -> iCmd/1
  Gain/1 -> Product/2
  %Flow vs Pot Position/1 -> Product1/1  "commanded_%flow"
  Pot position/1 -> %Flow vs Pot Position/1
  Percent Flow/1 -> Product1/2  "flow_reduction_%"
  Product1/1 -> Current versus %Flow/1  "%flow"
  Current versus %Flow/1 -> Terminator/1  "iCmd"

== stateflow chart MX_Gtwy_Control/System/Engine/Engine ==
  state NORMAL
    en:
    panelMissingDebounce= 0;
    flag_PrimeComplete = 0;
    state_TurboPrime = NORMAL;
    state CRANKING
      ex:
      state_TurboPrime = NORMAL;
      state FACTORY_TURBO_PRIMER
        state PREVENT_FUEL_MSG_ACTIVE
          en:
          state_Engine = ENGINE_CRANKING;
          SendMsg_03_Now();
          debouncePrimer = 0;
          du:
          SendMsg_DPM2_Now();
          debouncePrimer++;
          state_TurboPrime = ACTIVE;
        state CHECK_HOUR_METER
        state HOUR_METER_EXCEEDED
          en:
          state_Engine = ENGINE_CRANKING;
          SendMsg_03_Now();
        note: If hour meter is less than FACTORY_TURBO_PRIME_HOUR_ENABLE then send out message to prevent
            | ECU from providing fuel to the engine.  After 15 seconds, we need to stop cranking for 1 second so engine
            | speed can go to 0.  After, crank again but stop sending message such that fuel is allowed.
        state PREVENT_FUEL_MSG_INACTIVE
          en:
          state_Engine = ENGINE_CRANKING;
          SendMsg_03_Now();
        state PAUSE
          en:
          state_Engine = ENGINE_NOT_RUNNING;
          SendMsg_03_Now();
          debouncePrimer = 0;
          flag_PrimeComplete = 1;
          state_TurboPrime = NORMAL;
          du:
          debouncePrimer++;
          SendMsg_DPM2_Now();
        (default) -> CHECK_HOUR_METER
        (junction 26) -> PREVENT_FUEL_MSG_ACTIVE [order 1] : [CD_FACTORY_TURBO_PRIME_FUNCTION != NON_FUNCTIONAL...
            | && ((actualHourMeter <= CD_FACTORY_TURBO_PRIME_HOUR_ENABLE) || (dv_TurboPrime == TURBO_PRIME_ACTIVE))...
            | && !flag_PrimeComplete]
        CHECK_HOUR_METER -> (junction 26)
        (junction 26) -> HOUR_METER_EXCEEDED [order 2]
        PREVENT_FUEL_MSG_ACTIVE -> PAUSE : [(debouncePrimer >= CD_FACTORY_TURBO_PRIME_DEBOUNCE_DISABLE_FUEL)]
        PAUSE -> PREVENT_FUEL_MSG_INACTIVE : [(debouncePrimer >= CD_FACTORY_TURBO_PRIME_DEBOUNCE_WAIT)]
      (default) -> FACTORY_TURBO_PRIMER
    state NOT_RUNNING
      state NOT_RUNNING
        en:state_Engine = ENGINE_NOT_RUNNING;
        en:SendMsg_03_Now();
      state WAIT_TO_START
        en:state_Engine = ENGINE_INITIALIZE;
        en:debounceCount = 0;
        du:debounceCount++;
      (default) -> NORMAL.NOT_RUNNING.NOT_RUNNING
      WAIT_TO_START -> NORMAL.NOT_RUNNING.NOT_RUNNING : [((debounceCount >= CD_ECU_DEBOUNCE_WAIT_TO_START) && (state_CommECU == NORMAL))...
          | || (CD_ECU_FUNCTION == NON_FUNCTIONAL)]
    state RUNNING
      en:state_Engine = ENGINE_RUNNING;
      en:SendMsg_03_Now();
      RUNNING -> (junction 36) [order 1]
      (junction 36) -> (junction 37) [order 1] : [(CD_PANEL_SHUTDOWN_FUNCTION != NON_FUNCTIONAL) && (din_RemoteKey != ACTIVE)...
          | && ((state_CommStdPanel == NO_COMMUNICATION) || (state_DeluxeG5 == NO_COMMUNICATION) || (state_CommDeluxeG55 == NO_COMMUNICATION))]
      (junction 36) -> (junction 38) [order 2] : {
          | panelMissingDebounce= 0;
          | }
      (junction 37) -> (junction 39) : {
          | panelMissingDebounce++;
          | }
      (junction 39) -> (junction 38)
      (junction 38) -> (junction 40)
    NORMAL.NOT_RUNNING -> CRANKING [order 2] : [((state_EngineStart == ACTIVE))...
        | && (state_Key == ACTIVE)...
        | && (state_System == SYS_NORMAL)...
        | && ((CD_EMEA_CONSOLE_CRANK_INHIBIT == NON_FUNCTIONAL) || (state_Console == INACTIVE))...
        | && (((state_CommECU == NORMAL) && (ecu_EngineStarterMode != STARTER_INHIBITED) && (state_Engine == ENGINE_NOT_RUNNING))...
        |         || (CD_ECU_FUNCTION == NON_FUNCTIONAL))]
    CRANKING -> NORMAL.NOT_RUNNING [order 1] : [((state_EngineStart != ACTIVE))...
        | || (state_Key != ACTIVE)...
        | || (state_System != SYS_NORMAL)...
        | || ((CD_EMEA_CONSOLE_CRANK_INHIBIT != NON_FUNCTIONAL) && (state_Console != INACTIVE))...
        | || (ecu_EngineStarterMode == STARTER_INHIBITED)]
    NORMAL.NOT_RUNNING -> RUNNING [order 1] : [(flt_EngineSpeed >= CD_ENGINE_SPEED_RUNNING)...
        | && (state_System == SYS_NORMAL)]
    RUNNING -> NORMAL.NOT_RUNNING [order 2] : [((flt_EngineSpeed <= CD_ENGINE_SPEED_NOT_RUNNING)...
        | || (state_System != SYS_NORMAL))]
    CRANKING -> RUNNING [order 2] : [(flt_EngineSpeed >= CD_ENGINE_SPEED_RUNNING)]
    (default) -> WAIT_TO_START
  state ST_SHUTDOWN
    en:state_Engine = ENGINE_OFF;
    en:SendMsg_03_Now();
  state SHUTDOWN
    en:state_Engine = SHUTDOWN;
    en:SendMsg_03_Now();
  (default) -> NORMAL
  ST_SHUTDOWN -> NORMAL : [(can_ST_EStop == INACTIVE)...
      | && (can_ACD_EStop == INACTIVE)]
  NORMAL -> ST_SHUTDOWN [order 1] : [(can_ST_EStop == ACTIVE)...
      | || (can_ACD_EStop == ACTIVE)]
  RUNNING -> SHUTDOWN [order 1] : [(state_ECT == SHUTDOWN)...
      | || (state_EOP == SHUTDOWN)...
      | || (state_HOT == SHUTDOWN)...
      | || (state_EngineSpeed == SHUTDOWN)...
      | || (state_FuelTemp == SHUTDOWN)...
      | || (state_KeylessPanel == NO_COMMUNICATION)...
      | || (((state_CommECU == NO_COMMUNICATION) || (state_EngineSpeedDerate == SHUTDOWN)) && (CD_ECU_FUNCTION != NON_FUNCTIONAL))...
      | || (panelMissingDebounce > CD_PANEL_SHUTDOWN_DEBOUNCE)]
  NORMAL -> SHUTDOWN [order 2] : [(state_Key == MULTIPLE)]
  data: state_Engine output uint8; flt_EngineSpeed input uint16; state_EngineSpeed input uint8; state_ECT input uint8; state_EOP input uint8; state_HOT input uint8; state_EngineStart input uint8; CD_ENGINE_SPEED_RUNNING parameter uint16; CD_ENGINE_SPEED_NOT_RUNNING parameter uint16; state_Key input uint8; ACTIVE parameter uint8; ENGINE_CRANKING parameter uint8; ENGINE_NOT_RUNNING parameter uint8; ENGINE_RUNNING parameter uint8; INACTIVE parameter uint8; SHUTDOWN parameter uint8; state_System input uint8; SYS_NORMAL parameter uint8; NO_COMMUNICATION parameter uint8; CD_PANEL_SHUTDOWN_FUNCTION parameter uint8; NON_FUNCTIONAL parameter uint8; MULTIPLE parameter uint8; ENGINE_OFF parameter uint8; din_RemoteKey input uint8; NORMAL parameter uint8; state_KeylessPanel input uint8; CD_ECU_FUNCTION parameter uint8; state_CommECU input uint8; can_ST_EStop input uint8; can_ACD_EStop input uint8; ENGINE_INITIALIZE parameter uint8; CD_ECU_DEBOUNCE_WAIT_TO_START parameter uint16; debounceCount local uint16; STARTER_INHIBITED parameter uint8; ecu_EngineStarterMode input uint8; state_EngineSpeedDerate input uint8; state_FuelTemp input uint8; panelMissingDebounce local uint16; CD_PANEL_SHUTDOWN_DEBOUNCE parameter uint16; state_CommStdPanel input uint8; state_DeluxeG5 input uint8; state_CommDeluxeG55 input uint8; CD_EMEA_CONSOLE_CRANK_INHIBIT parameter uint8; state_Console input uint8; CD_FACTORY_TURBO_PRIME_FUNCTION parameter uint8; actualHourMeter input uint32; CD_FACTORY_TURBO_PRIME_HOUR_ENABLE parameter uint32; dv_TurboPrime input uint8; TURBO_PRIME_ACTIVE parameter uint8; debouncePrimer local uint16; CD_FACTORY_TURBO_PRIME_DEBOUNCE_DISABLE_FUEL parameter uint16; CD_FACTORY_TURBO_PRIME_DEBOUNCE_WAIT parameter uint16; flag_PrimeComplete local boolean; state_TurboPrime output uint8

== stateflow chart MX_Gtwy_Control/System/AutoIdle/Auto_Idle_Enable/Auto_Idle ==
  state Normal
    state NonFunctional
      en:
      state_AutoIdleEnable = INACTIVE;
    state On
      en:
      state_AutoIdleEnable = ACTIVE;
      ee_AutoIdleEnabled = ACTIVE;
      matlab_UpdateEEVar();
    state Off
      en:
      state_AutoIdleEnable = INACTIVE;
      ee_AutoIdleEnabled = INACTIVE;
      matlab_UpdateEEVar();
    (default) -> Normal.NonFunctional
    Normal.NonFunctional -> (junction 118) : [CD_AUTOIDLE_FUNCTION != NON_FUNCTIONAL...
        | && flag_InitEERead]
    (junction 118) -> Normal.On [order 1] : [ee_AutoIdleEnabled]
    (junction 118) -> Normal.Off [order 2]
    Normal.Off -> Normal.On : [AutoIdle_RisingEdge...
        |  && state_LoadSense == NORMAL]
    Normal.On -> Normal.Off [order 2] : [AutoIdle_RisingEdge]
    Normal.On -> Normal.Off [order 1] : [state_LoadSense != NORMAL]
  state Deluxe_G55
    state NonFunctional
      en:
      state_AutoIdleEnable = INACTIVE;
    state Normal
      state Off
        en:
        state_AutoIdleEnable = INACTIVE;
      state On
        en:
        state_AutoIdleEnable = ACTIVE;
      (default) -> Deluxe_G55.Normal.Off
      Deluxe_G55.Normal.Off -> Deluxe_G55.Normal.On : [dlx_G55_AutoIdleEnable == ACTIVE]
      Deluxe_G55.Normal.On -> Deluxe_G55.Normal.Off : [dlx_G55_AutoIdleEnable != ACTIVE]
    state Error
      en:
      state_AutoIdleEnable = IN_ERROR;
    (default) -> Deluxe_G55.NonFunctional
    Deluxe_G55.NonFunctional -> Deluxe_G55.Normal : [CD_AUTOIDLE_FUNCTION != NON_FUNCTIONAL]
    Deluxe_G55.Normal -> Error : [state_LoadSense != NORMAL]
    Error -> Deluxe_G55.Normal : [state_LoadSense == NORMAL]
  (default) -> (junction 146)
  (junction 146) -> Normal [order 1] : [CD_DELUXE_GEN5_5_FUNCTION == NON_FUNCTIONAL]
  (junction 146) -> Deluxe_G55 [order 2]
  data: state_AutoIdleEnable output uint8; AutoIdle_RisingEdge input uint8; ee_AutoIdleEnabled data_store_memory boolean; INACTIVE parameter uint8; ACTIVE parameter uint8; state_LoadSense input uint8; NORMAL parameter uint8; CD_AUTOIDLE_FUNCTION parameter uint8; NON_FUNCTIONAL parameter uint8; flag_InitEERead input boolean; dlx_G55_AutoIdleEnable input uint8; CD_DELUXE_GEN5_5_FUNCTION parameter uint8; IN_ERROR parameter uint8

== stateflow chart MX_Gtwy_Control/Inputs/SensorSupply1/Sensor_Supply1_State ==
  state NON_FUNCTIONAL
    en:state_SensorSupply1 = NORMAL;
  state OORH
    state Debounce
      en:debounceCount = 0;
      du:debounceCount++;
    state OORH
      en:state_SensorSupply1 = OORH;
    (default) -> OORH.Debounce
    OORH.Debounce -> OORH.OORH : [(debounceCount >= CD_SENSOR_SUPPLY1_DEBOUNCE_OORH)...
        | || (state_SensorSupply1 == OORH)]
  state NORMAL
    state Debounce
      en:debounceCount = 0;
      du:debounceCount++;
    state NORMAL
      en:state_SensorSupply1 = NORMAL;
    (default) -> NORMAL.Debounce
    NORMAL.Debounce -> NORMAL.NORMAL : [((debounceCount >= CD_SENSOR_SUPPLY1_DEBOUNCE_NORMAL)...
        | && (CD_SENSOR_SUPPLY1_DEBOUNCE_NORMAL != UNLIMITED))...
        | || (state_SensorSupply1 == NORMAL)]
  state OORL
    state Debounce
      en:debounceCount = 0;
      du:debounceCount++;
    state NORMAL
      en:state_SensorSupply1 = OORL;
    (default) -> OORL.Debounce
    OORL.Debounce -> OORL.NORMAL : [(debounceCount >= CD_SENSOR_SUPPLY1_DEBOUNCE_OORL)...
        | || (state_SensorSupply1 == OORL)]
  (default) -> NON_FUNCTIONAL
  NON_FUNCTIONAL -> NORMAL : [(CD_SENSOR_SUPPLY1_FUNCTION != NON_FUNCTIONAL)]
  NORMAL -> OORH [order 2] : [(ain_SensorSupply1 >= CD_SENSOR_SUPPLY1_OORH)]
  NORMAL -> OORL [order 1] : [(ain_SensorSupply1 <= CD_SENSOR_SUPPLY1_OORL)...
      | && (calc_VBattery >= CD_VBATTERY_LOW)]
  OORH -> NORMAL : [(ain_SensorSupply1 < CD_SENSOR_SUPPLY1_OORH)]
  OORL -> NORMAL : [(ain_SensorSupply1 > CD_SENSOR_SUPPLY1_OORL)...
      | || (calc_VBattery < CD_VBATTERY_LOW)]
  data: state_SensorSupply1 output uint8; ain_SensorSupply1 input uint16; CD_SENSOR_SUPPLY1_FUNCTION parameter uint8; CD_SENSOR_SUPPLY1_OORL parameter uint16; CD_SENSOR_SUPPLY1_OORH parameter uint16; CD_SENSOR_SUPPLY1_DEBOUNCE_NORMAL parameter uint16; CD_SENSOR_SUPPLY1_DEBOUNCE_OORH parameter uint16; CD_SENSOR_SUPPLY1_DEBOUNCE_OORL parameter uint16; debounceCount local uint16 =0; OORH parameter uint8; OORL parameter uint8; NON_FUNCTIONAL parameter uint8; NORMAL parameter uint8; UNLIMITED parameter uint16; calc_VBattery input fixdt(0,16,8); CD_VBATTERY_LOW parameter uint8

== stateflow chart MX_Gtwy_Control/Inputs/Load_Sense/Load_Sense/Pressure_Monitor ==
  state Invalid
    en:
    state_LoadSense = NORMAL;
  state Valid
    state Normal
      state Normal_DB
      state Normal
        en:
        state_LoadSense = NORMAL;
      (default) -> Normal_DB
      Normal_DB -> Valid.Normal.Normal : [after(CD_LOADSENSE_DB,tick) ||...
          |  state_LoadSense == NORMAL]
    state OORL_DB
    state OORL
      entry: state_LoadSense = OORL;
    state OORH_DB
    state OORH
      entry: state_LoadSense = OORH;
    (default) -> Valid.Normal
    Valid.Normal -> OORL_DB [order 1] : [volts_LoadSense < CD_LOADSENSE_OORL]
    OORL_DB -> OORL [order 1] : [after(CD_LOADSENSE_DB ,tick)]
    OORL_DB -> Valid.Normal [order 2] : [volts_LoadSense >= CD_LOADSENSE_OORL]
    OORL -> Valid.Normal : [volts_LoadSense >= CD_LOADSENSE_OORL]
    Valid.Normal -> OORH_DB [order 2] : [volts_LoadSense > CD_LOADSENSE_OORH]
    OORH_DB -> OORH [order 1] : [after(CD_LOADSENSE_DB ,tick)]
    OORH_DB -> Valid.Normal [order 2] : [volts_LoadSense <= CD_LOADSENSE_OORH]
    OORH -> Valid.Normal : [volts_LoadSense <= CD_LOADSENSE_OORH]
  (default) -> Invalid
  Invalid -> Valid : [state_SensorSupply == NORMAL]
  Valid -> Invalid : [state_SensorSupply != NORMAL]
  data: volts_LoadSense input uint8; state_LoadSense output uint8; CD_LOADSENSE_OORH parameter uint8; CD_LOADSENSE_OORL parameter uint8; CD_LOADSENSE_DB parameter uint8; NORMAL parameter uint8; OORH parameter uint8; OORL parameter uint8; state_SensorSupply input uint8

== stateflow chart MX_Gtwy_Control/System/ElectricAutomation/Chart1 ==
  note: This chart manage the status of the inverter.
      | There are the following states :
      | CONNECTED : the CPU is able to communicate on the CAN bus with the inverter
      | PRE OPERATIONAL : the CPU read the node but main contactor is open
      | OPERATIONAL : the main contactor is on but the out is off
      | FWD : ready to run forward
      | REV : ready to run reverse
      | STOP : there is a request of fast stop
  state STOP
    en:cmdInverter = CMD_INVERTER_GOTO_STOP;
    du:cmdInverter = CMD_INVERTER_GOTO_STOP;
    ex:cmdInverter = CMD_INVERTER_GOTO_STOP;
  state CONNECTED
    en:cmdInverter = CMD_INVERTER_GOTO_OPERATIONAL;
    du:cmdInverter = CMD_INVERTER_GOTO_OPERATIONAL;
    ex:cmdInverter = CMD_INVERTER_GOTO_OPERATIONAL;
  state OPERATIONAL
    en:cmdInverter = CMD_INVERTER_GOTO_READY;
    du:cmdInverter = CMD_INVERTER_GOTO_READY;
    du:cmdInverter = CMD_INVERTER_GOTO_READY;
    ex:targetSpeed = 0
  note: In this state the inverter is ready to run, is missing only the direction command when received the inverter will start at the target speed
  state READY
    en:cmdInverter = CMD_INVERTER_RUN_FWD;
    en:targetSpeed = 0;
    du:cmdInverter = CMD_INVERTER_RUN_FWD;
    du:targetSpeed = 0;
    ex:cmdInverter = CMD_INVERTER_RUN_FWD;
    ex:targetSpeed = 0;
  note: If received message from the inverter show that the inverter is in OPERATIONAL state set the status as OPERATIONAL else send the command to go in this state
  state RUN_FWD
    en:cmdInverter = CMD_INVERTER_RUN_FWD;
    en:targetSpeed = 0;
    du:cmdInverter = CMD_INVERTER_RUN_FWD;
    du:targetSpeed = 10 * in_Speed;
    ex:cmdInverter = CMD_INVERTER_RUN_FWD;
    ex:targetSpeed = 10 * in_Speed;
  (default) -> STOP
  STOP -> CONNECTED : [state_Inverter >= INVERTER_CONNECTED ]
  CONNECTED -> STOP [order 2] : [state_Inverter <= INVERTER_OPERATIONAL  || ~din_RunEnter]
  CONNECTED -> OPERATIONAL [order 1] : [state_Inverter >= INVERTER_OPERATIONAL && din_RunEnter]
  OPERATIONAL -> CONNECTED [order 2] : [state_Inverter < INVERTER_OPERATIONAL  || ~din_RunEnter]
  OPERATIONAL -> READY [order 1] : [state_Inverter >= INVERTER_READY && din_RunEnter]
  READY -> OPERATIONAL [order 2] : [state_Inverter < INVERTER_READY  || ~din_RunEnter]
  READY -> RUN_FWD [order 1] : [state_Inverter >= INVERTER_RUN_FWD  && din_RunEnter ]
  RUN_FWD -> READY : [state_Inverter < INVERTER_RUN_FWD  || ~din_RunEnter]
  data: cmdInverter output uint8; state_Inverter input double; din_RunEnter input double; targetSpeed output uint16; INVERTER_CONNECTED parameter double; INVERTER_OPERATIONAL parameter double; INVERTER_READY parameter double; CMD_INVERTER_GOTO_OPERATIONAL parameter double; CMD_INVERTER_RUN_REV parameter double; CMD_INVERTER_RUN_FWD parameter double; CMD_INVERTER_GOTO_READY parameter double; CMD_INVERTER_GOTO_STOP parameter double; in_Speed input double; INVERTER_RUN_FWD parameter double; INVERTER_STOP parameter double
